simulink model "v13_aa_rii_16r128dr_ver104"  (Simulink 7.9)
settings: Solver=FixedStepDiscrete  SolverMode=SingleTasking  StartTime=0.0  StopTime=32768  RelTol=1e-3
undefined symbols (not defined in the file or in its folder): sfp
libraries: xbsIndex_r4 x19795, xps_library x54, casper_library_misc x36, dspsigops x32
(part 6 of 212)

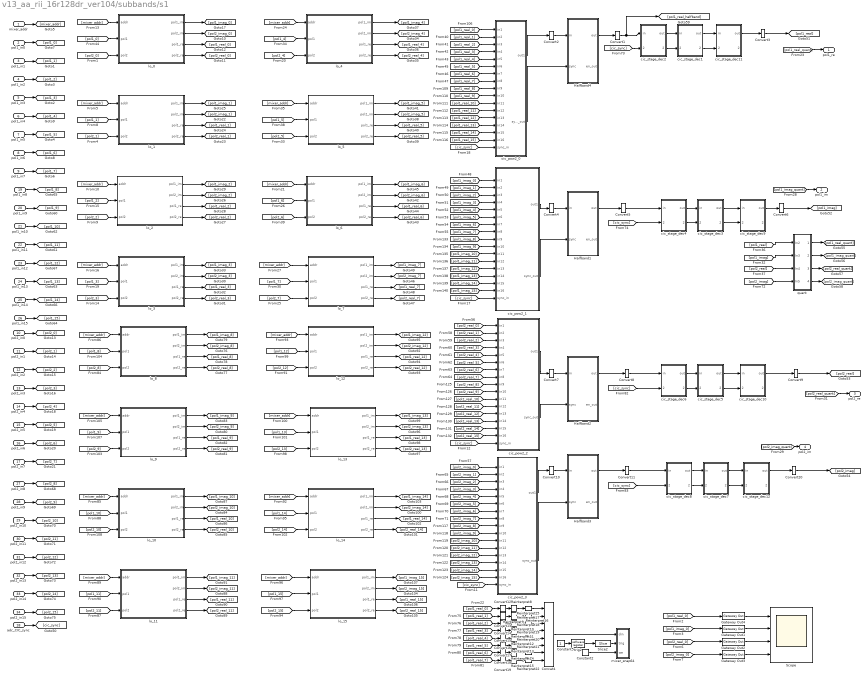
== system v13_aa_rii_16r128dr_ver104/subbands/s1 ==
  mixer_addr : Inport
  pol1_in0 : Inport  Port=2
  pol1_in1 : Inport  Port=3
  pol1_in2 : Inport  Port=4
  pol1_in3 : Inport  Port=5
  pol1_in4 : Inport  Port=6
  pol1_in5 : Inport  Port=7
  pol1_in6 : Inport  Port=8
  pol1_in7 : Inport  Port=9
  pol2_in0 : Inport  Port=10
  pol2_in1 : Inport  Port=11
  pol2_in2 : Inport  Port=12
  pol2_in3 : Inport  Port=13
  pol2_in4 : Inport  Port=14
  pol2_in5 : Inport  Port=15
  pol2_in6 : Inport  Port=16
  pol2_in7 : Inport  Port=17
  adc_CIC_sync : Inport  Port=18
  pol1_in8 : Inport  Port=19
  pol1_in9 : Inport  Port=20
  pol1_in10 : Inport  Port=21
  pol1_in11 : Inport  Port=22
  pol1_in12 : Inport  Port=23
  pol1_in13 : Inport  Port=24
  pol1_in14 : Inport  Port=25
  pol1_in15 : Inport  Port=26
  pol2_in8 : Inport  Port=27
  pol2_in9 : Inport  Port=28
  pol2_in10 : Inport  Port=29
  pol2_in11 : Inport  Port=30
  pol2_in12 : Inport  Port=31
  pol2_in13 : Inport  Port=32
  pol2_in14 : Inport  Port=33
  pol2_in15 : Inport  Port=34
  Concat4 : xbsIndex_r4/Concat  num_inputs=8  dbl_ovrd=off  block_type=concat
  Constant2 : xbsIndex_r4/Constant  const=1  gui_display_data_type=Boolean  arith_type=Boolean  n_bits=16  bin_pt=14  preci_type=Single  exp_width=8  frac_width=24  explicit_period=off  period=1  equ=P=C  opselect=C  inp2=PCIN>>17  opr=+  inp1=P  carry=CIN  dbl_ovrd=off  block_type=constant
  Constant5 : Constant
  Convert{1,5,8,11} (4 alike) : xbsIndex_r4/Convert  gui_display_data_type=Fixed-point  arith_type="Signed  (2's comp)"  n_bits=32  bin_pt=12  float_type=Single  exp_bits=8  fraction_bits=24  quantization=Truncate  overflow=Wrap  en=off  latency=1  dbl_ovrd=off  pipeline=off  block_type=convert
  Convert{2,4,7,10} (4 alike) : xbsIndex_r4/Convert  gui_display_data_type=Fixed-point  arith_type="Signed  (2's comp)"  n_bits=32  bin_pt=18  float_type=Single  exp_bits=8  fraction_bits=24  quantization=Truncate  overflow=Wrap  en=off  latency=1  dbl_ovrd=off  pipeline=off  block_type=convert
  Convert{12..19} (8 alike) : xbsIndex_r4/Convert  gui_display_data_type=Fixed-point  arith_type=Unsigned  n_bits=8  bin_pt=6  float_type=Single  exp_bits=8  fraction_bits=24  quantization=Truncate  overflow=Wrap  en=off  latency=2  dbl_ovrd=off  pipeline=off  block_type=convert
      settings: block_version=11.4
  Convert{3,6,9,20} (4 alike) : xbsIndex_r4/Convert  gui_display_data_type=Fixed-point  arith_type="Signed  (2's comp)"  n_bits=32  bin_pt=6  float_type=Single  exp_bits=8  fraction_bits=24  quantization=Truncate  overflow=Wrap  en=off  latency=1  dbl_ovrd=off  pipeline=off  block_type=convert
  Gateway Out{1..4} (4 alike) : xbsIndex_r4/Gateway Out  inherit_from_input=off  hdl_port=on  timing_constraint=None  locs_specified=off  LOCs={}  UseAsDAC=off  DACChannel='1'  block_type=gatewayout
      settings: infoedit="Gateway out block.  Converts Xilinx fixed point or floating-point type inputs into ouputs of type Simulink integer, double, or fixed point.<br><br>Hardware notes:  In hardware these blocks become top level output ports or are discarded, depending on how they are configured."
  Halfband1 : Subsystem  AncestorBlock=gbt_library/Halfband  mask(Halfband FIR){mult_latency=3, add_latency=2, hold_period=1, passband=0.4, fir_len=7}
      mask prompts: mult_latency "Multiplication latency"; add_latency "Add latency"; hold_period "Hold Period"; passband "Width of Halfband passband (<0.5)"; fir_len "FIR Length (odd) [Number of Taps will be: 2*fir_len+1]"
      mask help: A halfband FIR for low rate computation.
      mask init:
      | coeffs = halfband_coeff_gen(fir_len, passband);
      | config.source=str2func('hold_fir_init_xblock');
      | config.depend=[get_dependlist('hold_fir'),{'halfband_coeff_gen'}];
      | config.toplevel=gcb;
      | set_param(gcb,'LinkStatus','inactive');
      | xBlock(config, {config.toplevel,...
      |     'mult_latency', mult_latency, ...
      |     'add_latency', add_latency, ...
      |     'hold_period', hold_period, ...
      |     'coeffs', coeffs});
      settings: AttributesFormatString=|
      | 15-tap FIR filter (9 nonzero tap)
      |  hold period: 1
      |  mult latency: 3
      |  add latency: 2
  Halfband2 : Subsystem  AncestorBlock=gbt_library/Halfband  mask(Halfband FIR){mult_latency=3, add_latency=2, hold_period=1, passband=0.4, fir_len=7}
      mask prompts: mult_latency "Multiplication latency"; add_latency "Add latency"; hold_period "Hold Period"; passband "Width of Halfband passband (<0.5)"; fir_len "FIR Length (odd) [Number of Taps will be: 2*fir_len+1]"
      mask help: A halfband FIR for low rate computation.
      mask init: same as v13_aa_rii_16r128dr_ver104/subbands/s1/Halfband1
      settings: AttributesFormatString=|
      | 15-tap FIR filter (9 nonzero tap)
      |  hold period: 1
      |  mult latency: 3
      |  add latency: 2
  Halfband3 : Subsystem  AncestorBlock=gbt_library/Halfband  mask(Halfband FIR){mult_latency=3, add_latency=2, hold_period=1, passband=0.4, fir_len=7}
      mask prompts: mult_latency "Multiplication latency"; add_latency "Add latency"; hold_period "Hold Period"; passband "Width of Halfband passband (<0.5)"; fir_len "FIR Length (odd) [Number of Taps will be: 2*fir_len+1]"
      mask help: A halfband FIR for low rate computation.
      mask init: same as v13_aa_rii_16r128dr_ver104/subbands/s1/Halfband1
      settings: AttributesFormatString=|
      | 15-tap FIR filter (9 nonzero tap)
      |  hold period: 1
      |  mult latency: 3
      |  add latency: 2
  Halfband4 : Subsystem  AncestorBlock=gbt_library/Halfband  mask(Halfband FIR){mult_latency=3, add_latency=2, hold_period=1, passband=0.4, fir_len=7}
      mask prompts: mult_latency "Multiplication latency"; add_latency "Add latency"; hold_period "Hold Period"; passband "Width of Halfband passband (<0.5)"; fir_len "FIR Length (odd) [Number of Taps will be: 2*fir_len+1]"
      mask help: A halfband FIR for low rate computation.
      mask init: same as v13_aa_rii_16r128dr_ver104/subbands/s1/Halfband1
      settings: AttributesFormatString=|
      | 15-tap FIR filter (9 nonzero tap)
      |  hold period: 1
      |  mult latency: 3
      |  add latency: 2
  Reinterpret{8..15} (8 alike) : xbsIndex_r4/Reinterpret  force_arith_type=on  arith_type="Signed  (2's comp)"  force_bin_pt=on  bin_pt=7  block_type=reinterpret
  Reinterpret{16..22,25} (8 alike) : xbsIndex_r4/Reinterpret  force_arith_type=on  arith_type=Unsigned  force_bin_pt=on  bin_pt=0  block_type=reinterpret
  Scope : Scope  NumInputPorts=4
      settings: YMin=-0.0075~-1~-0.0075~-1  YMax=0.0075~1~0.0075~1
  Slice2 : xbsIndex_r4/Slice  nbits=1  boolean_output=on  mode=Lower Bit Location + Width  bit1=0  base1=MSB of Input  bit0=0  base0=LSB of Input  dbl_ovrd=off  block_type=slice
  cic_pow2_0 : Subsystem  AncestorBlock=gbt_library/cic_pow2  mask(CIC (pow2)){n_stages=4, dec_rate=16, input_clk_period=1, n_inputs=16, add_latency=1, n_bits=8, bin_pt=7}
      mask prompts: n_stages "CIC filter Order"; dec_rate "Decimation rate"; input_clk_period "Input Clock Period"; n_inputs "Number of inputs"; add_latency "Add latency"; n_bits "Input bit width"; bin_pt "Binary point"
      mask help:
      | A non-recursive CIC filter with parallel inputs.
      | Supports decimation rate of power of 2.
      | A multi-stage implementation with each stage of decimation rate 2.
      mask init:
      | config.source = str2func('cic_pow2_init_xblock');
      | config.toplevel = gcb;
      | config.depend = get_dependlist('cic_pow2'); 
      | set_param(gcb,'LinkStatus','inactive');
      | xBlock(config, {config.toplevel,...
      |                 'n_stages',n_stages, ...
      |                 'dec_rate', dec_rate, ...
      |                 'input_clk_rate', input_clk_period, ...
      |                 'n_inputs', n_inputs,...
      |                 'add_latency',add_latency,...
      |                 'n_bits', n_bits, ...
      |                 'bin_pt', bin_pt} ...
      |                 );
      | disp('new block added');
      settings: AttributesFormatString=|
      | dec_rate = 16
      | Order = 4
  cic_pow2_1 : Subsystem  AncestorBlock=gbt_library/cic_pow2  mask(CIC (pow2)){n_stages=4, dec_rate=16, input_clk_period=1, n_inputs=16, add_latency=1, n_bits=8, bin_pt=7}
      mask prompts: n_stages "CIC filter Order"; dec_rate "Decimation rate"; input_clk_period "Input Clock Period"; n_inputs "Number of inputs"; add_latency "Add latency"; n_bits "Input bit width"; bin_pt "Binary point"
      mask help:
      | A non-recursive CIC filter with parallel inputs.
      | Supports decimation rate of power of 2.
      | A multi-stage implementation with each stage of decimation rate 2.
      mask init: same as v13_aa_rii_16r128dr_ver104/subbands/s1/cic_pow2_0
      settings: AttributesFormatString=|
      | dec_rate = 16
      | Order = 4
  cic_pow2_2 : Subsystem  AncestorBlock=gbt_library/cic_pow2  mask(CIC (pow2)){n_stages=4, dec_rate=16, input_clk_period=1, n_inputs=16, add_latency=1, n_bits=8, bin_pt=7}
      mask prompts: n_stages "CIC filter Order"; dec_rate "Decimation rate"; input_clk_period "Input Clock Period"; n_inputs "Number of inputs"; add_latency "Add latency"; n_bits "Input bit width"; bin_pt "Binary point"
      mask help:
      | A non-recursive CIC filter with parallel inputs.
      | Supports decimation rate of power of 2.
      | A multi-stage implementation with each stage of decimation rate 2.
      mask init: same as v13_aa_rii_16r128dr_ver104/subbands/s1/cic_pow2_0
      settings: AttributesFormatString=|
      | dec_rate = 16
      | Order = 4
  cic_pow2_3 : Subsystem  AncestorBlock=gbt_library/cic_pow2  mask(CIC (pow2)){n_stages=4, dec_rate=16, input_clk_period=1, n_inputs=16, add_latency=1, n_bits=8, bin_pt=7}
      mask prompts: n_stages "CIC filter Order"; dec_rate "Decimation rate"; input_clk_period "Input Clock Period"; n_inputs "Number of inputs"; add_latency "Add latency"; n_bits "Input bit width"; bin_pt "Binary point"
      mask help:
      | A non-recursive CIC filter with parallel inputs.
      | Supports decimation rate of power of 2.
      | A multi-stage implementation with each stage of decimation rate 2.
      mask init: same as v13_aa_rii_16r128dr_ver104/subbands/s1/cic_pow2_0
      settings: AttributesFormatString=|
      | dec_rate = 16
      | Order = 4
  cic_stage_dec1 : Subsystem  AncestorBlock=gbt_library/cic_stage_dec2  mask(CIC stage (dec2)){n_stages=3, recursive=off, dec_rate=2, n_inputs=1, input_clk_period=1, input_period=2, add_latency=2, n_bits=8, bin_pt=7}
      mask prompts: n_stages "CIC filter Order"; recursive "Recursive?"; dec_rate "Decimation rate"; n_inputs "Number of Inputs"; input_clk_period "Input Clock period (Actual)"; input_period "Effective Input Sampling Period"; add_latency "Add latency"; n_bits "Input bit width"; bin_pt "Binary point"
      mask help:
      | A single stage of CIC filter with even number of parallel inputs.
      | Only supports decimation rate = 2.
      | If n_inputs > 1, number of outputs = n_inputs/2.
      | Non-recursive.
      |
      | Clock period:
      | input_clk_period is the actual sampling period of incoming data stream.
      | input_period is the effective (or expected) sampling period of incoming data stream.
      | E.g. For incoming data stream X[n] = [1& 1 2 2 3 3 4 4 5 5 ...], the input_clk_period is 1 and the input_period is 2.
      mask init:
      | if recursive
      |     % recursive case
      |     config.source=str2func('cic_stage_dec2_recursive_init_xblock');
      |     config.toplevel=gcb;
      |     config.depend = get_dependlist('cic_stage_dec2_recursive');
      |     set_param(gcb,'LinkStatus','inactive');
      |     xBlock(config, {config.toplevel, ...
      |                 'n_stages', n_stages, ...
      |                 'dec_rate', dec_rate, ...
      |                 'n_inputs', n_inputs, ...
      |                 'input_clk_period', input_clk_period, ...
      |                 'input_period', input_period, ...
      |                 'add_latency', add_latency, ...
      |                 'input_bits', n_bits, ...
      |                 'bin_pt', bin_pt, ...
      |                 'delay_len', 1});
      | else
      |     % non-recursive case
      |     config.source=str2func('cic_stage_dec2_init_xblock');
      |     config.toplevel=gcb;
      |     config.depend = get_dependlist('cic_stage_dec2');
      |     set_param(gcb,'LinkStatus','inactive');
      |     xBlock(config, {config.toplevel, ...
      |                 'n_stages', n_stages, ...
      |                 'dec_rate', dec_rate, ...
      |                 'n_inputs', n_inputs, ...
      |                 'input_clk_period', input_clk_period, ...
      |                 'input_period', input_period, ...
      |                 'add_latency', add_latency, ...
      |                 'n_bits', n_bits, ...
      |                 'bin_pt', bin_pt});
      | end
      settings: AttributesFormatString=|
      | nonrecursive
      | 8_7_in
      | clock(actualy/effective): 1/2
      mask CallbackString:
      | ||n=get_param(gcb,'dec_rate');
      | if ~isempty(n)
      |     n = eval(n);
      |     if ~isprime(n) && n~=1
      |         errordlg('Only supports decimation rate that is a prime number or 1');
      |         set_param(gcb,'dec_rate','');
      |     end
      | end||||||
  cic_stage_dec10 : Subsystem  AncestorBlock=gbt_library/cic_stage_dec2  mask(CIC stage (dec2)){n_stages=3, recursive=off, dec_rate=2, n_inputs=1, input_clk_period=1, input_period=4, add_latency=2, n_bits=8, bin_pt=7}
      mask prompts: n_stages "CIC filter Order"; recursive "Recursive?"; dec_rate "Decimation rate"; n_inputs "Number of Inputs"; input_clk_period "Input Clock period (Actual)"; input_period "Effective Input Sampling Period"; add_latency "Add latency"; n_bits "Input bit width"; bin_pt "Binary point"
      mask help:
      | A single stage of CIC filter with even number of parallel inputs.
      | Only supports decimation rate = 2.
      | If n_inputs > 1, number of outputs = n_inputs/2.
      | Non-recursive.
      |
      | Clock period:
      | input_clk_period is the actual sampling period of incoming data stream.
      | input_period is the effective (or expected) sampling period of incoming data stream.
      | E.g. For incoming data stream X[n] = [1& 1 2 2 3 3 4 4 5 5 ...], the input_clk_period is 1 and the input_period is 2.
      mask init: same as v13_aa_rii_16r128dr_ver104/subbands/s1/cic_stage_dec1
      settings: AttributesFormatString=|
      | nonrecursive
      | 8_7_in
      | clock(actualy/effective): 1/4
      mask CallbackString:
      | ||n=get_param(gcb,'dec_rate');
      | if ~isempty(n)
      |     n = eval(n);
      |     if ~isprime(n) && n~=1
      |         errordlg('Only supports decimation rate that is a prime number or 1');
      |         set_param(gcb,'dec_rate','');
      |     end
      | end||||||
  cic_stage_dec11 : Subsystem  AncestorBlock=gbt_library/cic_stage_dec2  mask(CIC stage (dec2)){n_stages=3, recursive=off, dec_rate=2, n_inputs=1, input_clk_period=1, input_period=4, add_latency=2, n_bits=8, bin_pt=7}
      mask prompts: n_stages "CIC filter Order"; recursive "Recursive?"; dec_rate "Decimation rate"; n_inputs "Number of Inputs"; input_clk_period "Input Clock period (Actual)"; input_period "Effective Input Sampling Period"; add_latency "Add latency"; n_bits "Input bit width"; bin_pt "Binary point"
      mask help:
      | A single stage of CIC filter with even number of parallel inputs.
      | Only supports decimation rate = 2.
      | If n_inputs > 1, number of outputs = n_inputs/2.
      | Non-recursive.
      |
      | Clock period:
      | input_clk_period is the actual sampling period of incoming data stream.
      | input_period is the effective (or expected) sampling period of incoming data stream.
      | E.g. For incoming data stream X[n] = [1& 1 2 2 3 3 4 4 5 5 ...], the input_clk_period is 1 and the input_period is 2.
      mask init: same as v13_aa_rii_16r128dr_ver104/subbands/s1/cic_stage_dec1
      settings: AttributesFormatString=|
      | nonrecursive
      | 8_7_in
      | clock(actualy/effective): 1/4
      mask CallbackString:
      | ||n=get_param(gcb,'dec_rate');
      | if ~isempty(n)
      |     n = eval(n);
      |     if ~isprime(n) && n~=1
      |         errordlg('Only supports decimation rate that is a prime number or 1');
      |         set_param(gcb,'dec_rate','');
      |     end
      | end||||||
  cic_stage_dec12 : Subsystem  AncestorBlock=gbt_library/cic_stage_dec2  mask(CIC stage (dec2)){n_stages=3, recursive=off, dec_rate=2, n_inputs=1, input_clk_period=1, input_period=4, add_latency=2, n_bits=8, bin_pt=7}
      mask prompts: n_stages "CIC filter Order"; recursive "Recursive?"; dec_rate "Decimation rate"; n_inputs "Number of Inputs"; input_clk_period "Input Clock period (Actual)"; input_period "Effective Input Sampling Period"; add_latency "Add latency"; n_bits "Input bit width"; bin_pt "Binary point"
      mask help:
      | A single stage of CIC filter with even number of parallel inputs.
      | Only supports decimation rate = 2.
      | If n_inputs > 1, number of outputs = n_inputs/2.
      | Non-recursive.
      |
      | Clock period:
      | input_clk_period is the actual sampling period of incoming data stream.
      | input_period is the effective (or expected) sampling period of incoming data stream.
      | E.g. For incoming data stream X[n] = [1& 1 2 2 3 3 4 4 5 5 ...], the input_clk_period is 1 and the input_period is 2.
      mask init: same as v13_aa_rii_16r128dr_ver104/subbands/s1/cic_stage_dec1
      settings: AttributesFormatString=|
      | nonrecursive
      | 8_7_in
      | clock(actualy/effective): 1/4
      mask CallbackString:
      | ||n=get_param(gcb,'dec_rate');
      | if ~isempty(n)
      |     n = eval(n);
      |     if ~isprime(n) && n~=1
      |         errordlg('Only supports decimation rate that is a prime number or 1');
      |         set_param(gcb,'dec_rate','');
      |     end
      | end||||||
  cic_stage_dec2 : Subsystem  AncestorBlock=gbt_library/cic_stage_dec2  mask(CIC stage (dec2)){n_stages=3, recursive=off, dec_rate=2, n_inputs=1, input_clk_period=1, input_period=1, add_latency=2, n_bits=8, bin_pt=7}
      mask prompts: n_stages "CIC filter Order"; recursive "Recursive?"; dec_rate "Decimation rate"; n_inputs "Number of Inputs"; input_clk_period "Input Clock period (Actual)"; input_period "Effective Input Sampling Period"; add_latency "Add latency"; n_bits "Input bit width"; bin_pt "Binary point"
      mask help:
      | A single stage of CIC filter with even number of parallel inputs.
      | Only supports decimation rate = 2.
      | If n_inputs > 1, number of outputs = n_inputs/2.
      | Non-recursive.
      |
      | Clock period:
      | input_clk_period is the actual sampling period of incoming data stream.
      | input_period is the effective (or expected) sampling period of incoming data stream.
      | E.g. For incoming data stream X[n] = [1& 1 2 2 3 3 4 4 5 5 ...], the input_clk_period is 1 and the input_period is 2.
      mask init: same as v13_aa_rii_16r128dr_ver104/subbands/s1/cic_stage_dec1
      settings: AttributesFormatString=|
      | nonrecursive
      | 8_7_in
      | clock(actualy/effective): 1/1
      mask CallbackString:
      | ||n=get_param(gcb,'dec_rate');
      | if ~isempty(n)
      |     n = eval(n);
      |     if ~isprime(n) && n~=1
      |         errordlg('Only supports decimation rate that is a prime number or 1');
      |         set_param(gcb,'dec_rate','');
      |     end
      | end||||||
  cic_stage_dec3 : Subsystem  AncestorBlock=gbt_library/cic_stage_dec2  mask(CIC stage (dec2)){n_stages=3, recursive=off, dec_rate=2, n_inputs=1, input_clk_period=1, input_period=2, add_latency=2, n_bits=8, bin_pt=7}
      mask prompts: n_stages "CIC filter Order"; recursive "Recursive?"; dec_rate "Decimation rate"; n_inputs "Number of Inputs"; input_clk_period "Input Clock period (Actual)"; input_period "Effective Input Sampling Period"; add_latency "Add latency"; n_bits "Input bit width"; bin_pt "Binary point"
      mask help:
      | A single stage of CIC filter with even number of parallel inputs.
      | Only supports decimation rate = 2.
      | If n_inputs > 1, number of outputs = n_inputs/2.
      | Non-recursive.
      |
      | Clock period:
      | input_clk_period is the actual sampling period of incoming data stream.
      | input_period is the effective (or expected) sampling period of incoming data stream.
      | E.g. For incoming data stream X[n] = [1& 1 2 2 3 3 4 4 5 5 ...], the input_clk_period is 1 and the input_period is 2.
      mask init: same as v13_aa_rii_16r128dr_ver104/subbands/s1/cic_stage_dec1
      settings: AttributesFormatString=|
      | nonrecursive
      | 8_7_in
      | clock(actualy/effective): 1/2
      mask CallbackString:
      | ||n=get_param(gcb,'dec_rate');
      | if ~isempty(n)
      |     n = eval(n);
      |     if ~isprime(n) && n~=1
      |         errordlg('Only supports decimation rate that is a prime number or 1');
      |         set_param(gcb,'dec_rate','');
      |     end
      | end||||||
  cic_stage_dec4 : Subsystem  AncestorBlock=gbt_library/cic_stage_dec2  mask(CIC stage (dec2)){n_stages=3, recursive=off, dec_rate=2, n_inputs=1, input_clk_period=1, input_period=1, add_latency=2, n_bits=8, bin_pt=7}
      mask prompts: n_stages "CIC filter Order"; recursive "Recursive?"; dec_rate "Decimation rate"; n_inputs "Number of Inputs"; input_clk_period "Input Clock period (Actual)"; input_period "Effective Input Sampling Period"; add_latency "Add latency"; n_bits "Input bit width"; bin_pt "Binary point"
      mask help:
      | A single stage of CIC filter with even number of parallel inputs.
      | Only supports decimation rate = 2.
      | If n_inputs > 1, number of outputs = n_inputs/2.
      | Non-recursive.
      |
      | Clock period:
      | input_clk_period is the actual sampling period of incoming data stream.
      | input_period is the effective (or expected) sampling period of incoming data stream.
      | E.g. For incoming data stream X[n] = [1& 1 2 2 3 3 4 4 5 5 ...], the input_clk_period is 1 and the input_period is 2.
      mask init: same as v13_aa_rii_16r128dr_ver104/subbands/s1/cic_stage_dec1
      settings: AttributesFormatString=|
      | nonrecursive
      | 8_7_in
      | clock(actualy/effective): 1/1
      mask CallbackString:
      | ||n=get_param(gcb,'dec_rate');
      | if ~isempty(n)
      |     n = eval(n);
      |     if ~isprime(n) && n~=1
      |         errordlg('Only supports decimation rate that is a prime number or 1');
      |         set_param(gcb,'dec_rate','');
      |     end
      | end||||||
  cic_stage_dec5 : Subsystem  AncestorBlock=gbt_library/cic_stage_dec2  mask(CIC stage (dec2)){n_stages=3, recursive=off, dec_rate=2, n_inputs=1, input_clk_period=1, input_period=2, add_latency=2, n_bits=8, bin_pt=7}
      mask prompts: n_stages "CIC filter Order"; recursive "Recursive?"; dec_rate "Decimation rate"; n_inputs "Number of Inputs"; input_clk_period "Input Clock period (Actual)"; input_period "Effective Input Sampling Period"; add_latency "Add latency"; n_bits "Input bit width"; bin_pt "Binary point"
      mask help:
      | A single stage of CIC filter with even number of parallel inputs.
      | Only supports decimation rate = 2.
      | If n_inputs > 1, number of outputs = n_inputs/2.
      | Non-recursive.
      |
      | Clock period:
      | input_clk_period is the actual sampling period of incoming data stream.
      | input_period is the effective (or expected) sampling period of incoming data stream.
      | E.g. For incoming data stream X[n] = [1& 1 2 2 3 3 4 4 5 5 ...], the input_clk_period is 1 and the input_period is 2.
      mask init: same as v13_aa_rii_16r128dr_ver104/subbands/s1/cic_stage_dec1
      settings: AttributesFormatString=|
      | nonrecursive
      | 8_7_in
      | clock(actualy/effective): 1/2
      mask CallbackString:
      | ||n=get_param(gcb,'dec_rate');
      | if ~isempty(n)
      |     n = eval(n);
      |     if ~isprime(n) && n~=1
      |         errordlg('Only supports decimation rate that is a prime number or 1');
      |         set_param(gcb,'dec_rate','');
      |     end
      | end||||||
  cic_stage_dec6 : Subsystem  AncestorBlock=gbt_library/cic_stage_dec2  mask(CIC stage (dec2)){n_stages=3, recursive=off, dec_rate=2, n_inputs=1, input_clk_period=1, input_period=1, add_latency=2, n_bits=8, bin_pt=7}
      mask prompts: n_stages "CIC filter Order"; recursive "Recursive?"; dec_rate "Decimation rate"; n_inputs "Number of Inputs"; input_clk_period "Input Clock period (Actual)"; input_period "Effective Input Sampling Period"; add_latency "Add latency"; n_bits "Input bit width"; bin_pt "Binary point"
      mask help:
      | A single stage of CIC filter with even number of parallel inputs.
      | Only supports decimation rate = 2.
      | If n_inputs > 1, number of outputs = n_inputs/2.
      | Non-recursive.
      |
      | Clock period:
      | input_clk_period is the actual sampling period of incoming data stream.
      | input_period is the effective (or expected) sampling period of incoming data stream.
      | E.g. For incoming data stream X[n] = [1& 1 2 2 3 3 4 4 5 5 ...], the input_clk_period is 1 and the input_period is 2.
      mask init: same as v13_aa_rii_16r128dr_ver104/subbands/s1/cic_stage_dec1
      settings: AttributesFormatString=|
      | nonrecursive
      | 8_7_in
      | clock(actualy/effective): 1/1
      mask CallbackString:
      | ||n=get_param(gcb,'dec_rate');
      | if ~isempty(n)
      |     n = eval(n);
      |     if ~isprime(n) && n~=1
      |         errordlg('Only supports decimation rate that is a prime number or 1');
      |         set_param(gcb,'dec_rate','');
      |     end
      | end||||||
  cic_stage_dec7 : Subsystem  AncestorBlock=gbt_library/cic_stage_dec2  mask(CIC stage (dec2)){n_stages=3, recursive=off, dec_rate=2, n_inputs=1, input_clk_period=1, input_period=2, add_latency=2, n_bits=8, bin_pt=7}
      mask prompts: n_stages "CIC filter Order"; recursive "Recursive?"; dec_rate "Decimation rate"; n_inputs "Number of Inputs"; input_clk_period "Input Clock period (Actual)"; input_period "Effective Input Sampling Period"; add_latency "Add latency"; n_bits "Input bit width"; bin_pt "Binary point"
      mask help:
      | A single stage of CIC filter with even number of parallel inputs.
      | Only supports decimation rate = 2.
      | If n_inputs > 1, number of outputs = n_inputs/2.
      | Non-recursive.
      |
      | Clock period:
      | input_clk_period is the actual sampling period of incoming data stream.
      | input_period is the effective (or expected) sampling period of incoming data stream.
      | E.g. For incoming data stream X[n] = [1& 1 2 2 3 3 4 4 5 5 ...], the input_clk_period is 1 and the input_period is 2.
      mask init: same as v13_aa_rii_16r128dr_ver104/subbands/s1/cic_stage_dec1
      settings: AttributesFormatString=|
      | nonrecursive
      | 8_7_in
      | clock(actualy/effective): 1/2
      mask CallbackString:
      | ||n=get_param(gcb,'dec_rate');
      | if ~isempty(n)
      |     n = eval(n);
      |     if ~isprime(n) && n~=1
      |         errordlg('Only supports decimation rate that is a prime number or 1');
      |         set_param(gcb,'dec_rate','');
      |     end
      | end||||||
  cic_stage_dec8 : Subsystem  AncestorBlock=gbt_library/cic_stage_dec2  mask(CIC stage (dec2)){n_stages=3, recursive=off, dec_rate=2, n_inputs=1, input_clk_period=1, input_period=1, add_latency=2, n_bits=8, bin_pt=7}
      mask prompts: n_stages "CIC filter Order"; recursive "Recursive?"; dec_rate "Decimation rate"; n_inputs "Number of Inputs"; input_clk_period "Input Clock period (Actual)"; input_period "Effective Input Sampling Period"; add_latency "Add latency"; n_bits "Input bit width"; bin_pt "Binary point"
      mask help:
      | A single stage of CIC filter with even number of parallel inputs.
      | Only supports decimation rate = 2.
      | If n_inputs > 1, number of outputs = n_inputs/2.
      | Non-recursive.
      |
      | Clock period:
      | input_clk_period is the actual sampling period of incoming data stream.
      | input_period is the effective (or expected) sampling period of incoming data stream.
      | E.g. For incoming data stream X[n] = [1& 1 2 2 3 3 4 4 5 5 ...], the input_clk_period is 1 and the input_period is 2.
      mask init: same as v13_aa_rii_16r128dr_ver104/subbands/s1/cic_stage_dec1
      settings: AttributesFormatString=|
      | nonrecursive
      | 8_7_in
      | clock(actualy/effective): 1/1
      mask CallbackString:
      | ||n=get_param(gcb,'dec_rate');
      | if ~isempty(n)
      |     n = eval(n);
      |     if ~isprime(n) && n~=1
      |         errordlg('Only supports decimation rate that is a prime number or 1');
      |         set_param(gcb,'dec_rate','');
      |     end
      | end||||||
  cic_stage_dec9 : Subsystem  AncestorBlock=gbt_library/cic_stage_dec2  mask(CIC stage (dec2)){n_stages=3, recursive=off, dec_rate=2, n_inputs=1, input_clk_period=1, input_period=4, add_latency=2, n_bits=8, bin_pt=7}
      mask prompts: n_stages "CIC filter Order"; recursive "Recursive?"; dec_rate "Decimation rate"; n_inputs "Number of Inputs"; input_clk_period "Input Clock period (Actual)"; input_period "Effective Input Sampling Period"; add_latency "Add latency"; n_bits "Input bit width"; bin_pt "Binary point"
      mask help:
      | A single stage of CIC filter with even number of parallel inputs.
      | Only supports decimation rate = 2.
      | If n_inputs > 1, number of outputs = n_inputs/2.
      | Non-recursive.
      |
      | Clock period:
      | input_clk_period is the actual sampling period of incoming data stream.
      | input_period is the effective (or expected) sampling period of incoming data stream.
      | E.g. For incoming data stream X[n] = [1& 1 2 2 3 3 4 4 5 5 ...], the input_clk_period is 1 and the input_period is 2.
      mask init: same as v13_aa_rii_16r128dr_ver104/subbands/s1/cic_stage_dec1
      settings: AttributesFormatString=|
      | nonrecursive
      | 8_7_in
      | clock(actualy/effective): 1/4
      mask CallbackString:
      | ||n=get_param(gcb,'dec_rate');
      | if ~isempty(n)
      |     n = eval(n);
      |     if ~isprime(n) && n~=1
      |         errordlg('Only supports decimation rate that is a prime number or 1');
      |         set_param(gcb,'dec_rate','');
      |     end
      | end||||||
  lo_0 : Subsystem  AncestorBlock=gbt_library/complex_mix_lo
  lo_1 : Subsystem  AncestorBlock=gbt_library/complex_mix_lo
  lo_10 : Subsystem  AncestorBlock=gbt_library/complex_mix_lo
  lo_11 : Subsystem  AncestorBlock=gbt_library/complex_mix_lo
  lo_12 : Subsystem  AncestorBlock=gbt_library/complex_mix_lo
  lo_13 : Subsystem  AncestorBlock=gbt_library/complex_mix_lo
  lo_14 : Subsystem  AncestorBlock=gbt_library/complex_mix_lo
  lo_15 : Subsystem  AncestorBlock=gbt_library/complex_mix_lo
  lo_2 : Subsystem  AncestorBlock=gbt_library/complex_mix_lo
  lo_3 : Subsystem  AncestorBlock=gbt_library/complex_mix_lo
  lo_4 : Subsystem  AncestorBlock=gbt_library/complex_mix_lo
  lo_5 : Subsystem  AncestorBlock=gbt_library/complex_mix_lo
  lo_6 : Subsystem  AncestorBlock=gbt_library/complex_mix_lo
  lo_7 : Subsystem  AncestorBlock=gbt_library/complex_mix_lo
  lo_8 : Subsystem  AncestorBlock=gbt_library/complex_mix_lo
  lo_9 : Subsystem  AncestorBlock=gbt_library/complex_mix_lo
  mixer_snap64 : Subsystem  AncestorBlock=casper_library_scopes/snap64  mask(snap64){nsamples=11}
      mask prompts: nsamples "No. of samples(2^?)"
      mask help:
      | The <i>snap</i> block provides a packaged solution to capturing data from
      | the FPGA fabric and making it accessible from the CPU.  The block comes in
      | 2 flavors: <i>snap</i> (which captures to a 32 bit wide Shared BRAM), and
      | <i>snap64</i> (which captures to 2x32 bit wide Shared BRAMs to effect a
      | 64 bit capture).
      mask Help: eval('xlWeb(''http://casper.berkeley.edu/wiki/Snap'')')
  quant : Subsystem
  trig2 : xps_library/software register  Tag=xps:sw_reg  io_dir=From Processor  arith_type=Unsigned  bitwidth=32  bin_pt=0  sample_period=1  gw_name=v13_aa_rii_16r128dr_ver104_subbands_s1_trig2_user_data_out
      link override cic_filter_testing_to_compile_trig_user_data_out: arith_type=Unsigned  n_bits=32  bin_pt=0  period=1
      link override newmode13_subband1_trig2_user_data_out: arith_type=Unsigned  n_bits=32  bin_pt=0  period=1
  pol1_re : Outport
  pol1_im : Outport  Port=2
  pol2_re : Outport  Port=3
  pol2_im : Outport  Port=4
  wires:
  mixer_addr/1 -> [mixer_addr]
  pol1_in0/1 -> [pol1_0]
  pol1_in1/1 -> [pol1_1]
  pol1_in2/1 -> [pol1_2]
  pol1_in3/1 -> [pol1_3]
  pol1_in4/1 -> [pol1_4]
  pol1_in5/1 -> [pol1_5]
  pol1_in6/1 -> [pol1_6]
  pol1_in7/1 -> [pol1_7]
  pol2_in0/1 -> [pol2_0]
  pol2_in1/1 -> [pol2_1]
  pol2_in2/1 -> [pol2_2]
  pol2_in3/1 -> [pol2_3]
  pol2_in4/1 -> [pol2_4]
  pol2_in5/1 -> [pol2_5]
  pol2_in6/1 -> [pol2_6]
  pol2_in7/1 -> [pol2_7]
  [mixer_addr] -> lo_1/1(addr)
  [mixer_addr] -> lo_2/1(addr)
  [mixer_addr] -> lo_3/1(addr)
  lo_4/1(pol1_im) -> [pol1_imag_4]
  lo_4/2(pol2_im) -> [pol2_imag_4]
  lo_4/3(pol1_re) -> [pol1_real_4]
  lo_4/4(pol2_re) -> [pol2_real_4]
  [mixer_addr] -> lo_4/1(addr)
  [pol2_4] -> lo_4/3(pol2)
  [pol1_4] -> lo_4/2(pol1)
  [mixer_addr] -> lo_0/1(addr)
  [pol1_0] -> lo_0/2(pol1)
  [pol2_0] -> lo_0/3(pol2)
  lo_0/1(pol1_im) -> [pol1_imag_0]
  lo_0/2(pol2_im) -> [pol2_imag_0]
  lo_0/3(pol1_re) -> [pol1_real_0]
  lo_0/4(pol2_re) -> [pol2_real_0]
  [pol1_1] -> lo_1/2(pol1)
  [pol2_1] -> lo_1/3(pol2)
  lo_1/1(pol1_im) -> [pol1_imag_1]
  lo_1/2(pol2_im) -> [pol2_imag_1]
  lo_1/3(pol1_re) -> [pol1_real_1]
  lo_1/4(pol2_re) -> [pol2_real_1]
  [pol1_2] -> lo_2/2(pol1)
  [pol2_2] -> lo_2/3(pol2)
  [pol1_3] -> lo_3/2(pol1)
  [pol2_3] -> lo_3/3(pol2)
  lo_3/1(pol1_im) -> [pol1_imag_3]
  lo_3/2(pol2_im) -> [pol2_imag_3]
  lo_3/3(pol1_re) -> [pol1_real_3]
  lo_3/4(pol2_re) -> [pol2_real_3]
  [mixer_addr] -> lo_5/1(addr)
  [pol1_5] -> lo_5/2(pol1)
  [pol2_5] -> lo_5/3(pol2)
  lo_5/1(pol1_im) -> [pol1_imag_5]
  lo_5/2(pol2_im) -> [pol2_imag_5]
  lo_5/3(pol1_re) -> [pol1_real_5]
  lo_5/4(pol2_re) -> [pol2_real_5]
  [mixer_addr] -> lo_6/1(addr)
  [pol1_6] -> lo_6/2(pol1)
  [pol2_6] -> lo_6/3(pol2)
  lo_6/1(pol1_im) -> [pol1_imag_6]
  lo_6/2(pol2_im) -> [pol2_imag_6]
  lo_6/3(pol1_re) -> [pol1_real_6]
  lo_6/4(pol2_re) -> [pol2_real_6]
  [mixer_addr] -> lo_7/1(addr)
  [pol1_7] -> lo_7/2(pol1)
  [pol2_7] -> lo_7/3(pol2)
  lo_7/1(pol1_im) -> [pol1_imag_7]
  lo_7/2(pol2_im) -> [pol2_imag_7]
  lo_7/3(pol1_re) -> [pol1_real_7]
  lo_7/4(pol2_re) -> [pol2_real_7]
  lo_2/1(pol1_im) -> [pol1_imag_2]
  lo_2/2(pol2_im) -> [pol2_imag_2]
  lo_2/3(pol1_re) -> [pol1_real_2]
  lo_2/4(pol2_re) -> [pol2_real_2]
  [pol1_real_0] -> Gateway Out4/1
  [pol1_imag_0] -> Gateway Out1/1
  [pol2_real_0] -> Gateway Out2/1
  [pol2_imag_0] -> Gateway Out3/1
  Gateway Out4/1 -> Scope/1
  Gateway Out1/1 -> Scope/2
  Gateway Out2/1 -> Scope/3
  Gateway Out3/1 -> Scope/4
  adc_CIC_sync/1 -> [cic_sync]
  Concat4/1 -> mixer_snap64/1(din)
  Constant2/1 -> mixer_snap64/3(we)
  Convert12/1 -> Reinterpret8/1
  [pol1_real_0] -> Convert12/1
  Reinterpret8/1 -> Reinterpret25/1
  Convert13/1 -> Reinterpret9/1
  [pol1_real_1] -> Convert13/1
  Reinterpret9/1 -> Reinterpret16/1
  Convert14/1 -> Reinterpret10/1
  [pol1_real_2] -> Convert14/1
  Reinterpret10/1 -> Reinterpret17/1
  Convert15/1 -> Reinterpret11/1
  [pol1_real_3] -> Convert15/1
  Reinterpret11/1 -> Reinterpret18/1
  Convert16/1 -> Reinterpret12/1
  [pol1_real_4] -> Convert16/1
  Reinterpret12/1 -> Reinterpret19/1
  Convert17/1 -> Reinterpret13/1
  [pol1_real_5] -> Convert17/1
  Reinterpret13/1 -> Reinterpret20/1
  Convert18/1 -> Reinterpret14/1
  [pol1_real_6] -> Convert18/1
  Reinterpret14/1 -> Reinterpret21/1
  Convert19/1 -> Reinterpret15/1
  [pol1_real_7] -> Convert19/1
  Reinterpret15/1 -> Reinterpret22/1
  Reinterpret25/1 -> Concat4/1
  Reinterpret16/1 -> Concat4/2
  Reinterpret17/1 -> Concat4/3
  Reinterpret18/1 -> Concat4/4
  Reinterpret19/1 -> Concat4/5
  Reinterpret20/1 -> Concat4/6
  Reinterpret21/1 -> Concat4/7
  Reinterpret22/1 -> Concat4/8
  Constant5/1 -> trig2/1
  trig2/1 -> Slice2/1
  Slice2/1 -> mixer_snap64/2(trig)
  [pol1_real_quant] -> pol1_re/1
  cic_pow2_0/2(sync_out) -> Halfband4/2(sync)
  cic_stage_dec2/2(sync_out) -> cic_stage_dec1/2(sync_in)
  cic_stage_dec2/1(out) -> cic_stage_dec1/1(in)
  Halfband4/1(out) -> Convert1/1
  [cic_sync] -> cic_stage_dec2/2(sync_in)
  cic_pow2_0/1(out1) -> Convert2/1
  Convert2/1 -> Halfband4/1(in)
  Convert1/1 -> cic_stage_dec2/1(in), [pol1_real_halfband]
  Convert3/1 -> [pol1_real]
  [pol1_imag_quant] -> pol1_im/1
  quant/1(Out1) -> [pol1_real_quant]
  quant/2(Out2) -> [pol1_imag_quant]
  [pol1_real] -> quant/1(In2)
  [pol1_imag] -> quant/2(In3)
  cic_stage_dec4/2(sync_out) -> cic_stage_dec3/2(sync_in)
  cic_stage_dec4/1(out) -> cic_stage_dec3/1(in)
  Halfband1/1(out) -> Convert5/1
  [cic_sync] -> cic_stage_dec4/2(sync_in)
  cic_pow2_1/1(out1) -> Convert4/1
  cic_pow2_1/2(sync_out) -> Halfband1/2(sync)
  Convert4/1 -> Halfband1/1(in)
  Convert5/1 -> cic_stage_dec4/1(in)
  Convert6/1 -> [pol1_imag]
  [pol2_real] -> quant/3(In4)
  [pol2_imag] -> quant/4(In5)
  quant/3(Out3) -> [pol2_real_quant]
  quant/4(Out4) -> [pol2_imag_quant]
  [pol2_real_quant] -> pol2_re/1
  cic_stage_dec6/2(sync_out) -> cic_stage_dec5/2(sync_in)
  cic_stage_dec6/1(out) -> cic_stage_dec5/1(in)
  Halfband2/1(out) -> Convert8/1
  [cic_sync] -> cic_stage_dec6/2(sync_in)
  cic_pow2_2/1(out1) -> Convert7/1
  cic_pow2_2/2(sync_out) -> Halfband2/2(sync)
  Convert7/1 -> Halfband2/1(in)
  Convert8/1 -> cic_stage_dec6/1(in)
  Convert9/1 -> [pol2_real]
  [pol2_imag_quant] -> pol2_im/1
  cic_stage_dec8/2(sync_out) -> cic_stage_dec7/2(sync_in)
  cic_stage_dec8/1(out) -> cic_stage_dec7/1(in)
  Halfband3/1(out) -> Convert11/1
  [cic_sync] -> cic_stage_dec8/2(sync_in)
  cic_pow2_3/1(out1) -> Convert10/1
  cic_pow2_3/2(sync_out) -> Halfband3/2(sync)
  Convert10/1 -> Halfband3/1(in)
  Convert11/1 -> cic_stage_dec8/1(in)
  Convert20/1 -> [pol2_imag]
  pol1_in9/1 -> [pol1_9]
  pol1_in10/1 -> [pol1_10]
  pol1_in11/1 -> [pol1_11]
  pol1_in12/1 -> [pol1_12]
  pol1_in13/1 -> [pol1_13]
  pol1_in14/1 -> [pol1_14]
  pol1_in15/1 -> [pol1_15]
  pol2_in8/1 -> [pol2_8]
  pol2_in9/1 -> [pol2_9]
  pol2_in10/1 -> [pol2_10]
  pol2_in11/1 -> [pol2_11]
  pol2_in12/1 -> [pol2_12]
  pol2_in13/1 -> [pol2_13]
  pol2_in14/1 -> [pol2_14]
  pol2_in15/1 -> [pol2_15]
  [mixer_addr] -> lo_9/1(addr)
  [mixer_addr] -> lo_10/1(addr)
  [mixer_addr] -> lo_11/1(addr)
  lo_12/1(pol1_im) -> [pol1_imag_12]
  lo_12/2(pol2_im) -> [pol2_imag_12]
  lo_12/3(pol1_re) -> [pol1_real_12]
  lo_12/4(pol2_re) -> [pol2_real_12]
  [mixer_addr] -> lo_12/1(addr)
  [pol2_12] -> lo_12/3(pol2)
  [pol1_12] -> lo_12/2(pol1)
  [mixer_addr] -> lo_8/1(addr)
  [pol1_8] -> lo_8/2(pol1)
  [pol2_8] -> lo_8/3(pol2)
  lo_8/1(pol1_im) -> [pol1_imag_8]
  lo_8/2(pol2_im) -> [pol2_imag_8]
  lo_8/3(pol1_re) -> [pol1_real_8]
  lo_8/4(pol2_re) -> [pol2_real_8]
  [pol1_9] -> lo_9/2(pol1)
  [pol2_9] -> lo_9/3(pol2)
  lo_9/1(pol1_im) -> [pol1_imag_9]
  lo_9/2(pol2_im) -> [pol2_imag_9]
  lo_9/3(pol1_re) -> [pol1_real_9]
  lo_9/4(pol2_re) -> [pol2_real_9]
  [pol1_10] -> lo_10/2(pol1)
  [pol2_10] -> lo_10/3(pol2)
  [pol1_11] -> lo_11/2(pol1)
  [pol2_11] -> lo_11/3(pol2)
  lo_11/1(pol1_im) -> [pol1_imag_11]
  lo_11/2(pol2_im) -> [pol2_imag_11]
  lo_11/3(pol1_re) -> [pol1_real_11]
  lo_11/4(pol2_re) -> [pol2_real_11]
  [mixer_addr] -> lo_13/1(addr)
  [pol1_13] -> lo_13/2(pol1)
  [pol2_13] -> lo_13/3(pol2)
  lo_13/1(pol1_im) -> [pol1_imag_13]
  lo_13/2(pol2_im) -> [pol2_imag_13]
  lo_13/3(pol1_re) -> [pol1_real_13]
  lo_13/4(pol2_re) -> [pol2_real_13]
  [mixer_addr] -> lo_14/1(addr)
  [pol1_14] -> lo_14/2(pol1)
  [pol2_14] -> lo_14/3(pol2)
  lo_14/1(pol1_im) -> [pol1_imag_14]
  lo_14/2(pol2_im) -> [pol2_imag_14]
  lo_14/3(pol1_re) -> [pol1_real_14]
  lo_14/4(pol2_re) -> [pol2_real_14]
  [mixer_addr] -> lo_15/1(addr)
  [pol1_15] -> lo_15/2(pol1)
  [pol2_15] -> lo_15/3(pol2)
  lo_15/1(pol1_im) -> [pol1_imag_15]
  lo_15/2(pol2_im) -> [pol2_imag_15]
  lo_15/3(pol1_re) -> [pol1_real_15]
  lo_15/4(pol2_re) -> [pol2_real_15]
  lo_10/1(pol1_im) -> [pol1_imag_10]
  lo_10/2(pol2_im) -> [pol2_imag_10]
  lo_10/3(pol1_re) -> [pol1_real_10]
  lo_10/4(pol2_re) -> [pol2_real_10]
  [cic_sync] -> cic_pow2_0/17(sync_in)
  [pol1_real_0] -> cic_pow2_0/1(in1)
  [pol1_real_1] -> cic_pow2_0/2(in2)
  [pol1_real_2] -> cic_pow2_0/3(in3)
  [pol1_real_3] -> cic_pow2_0/4(in4)
  [pol1_real_4] -> cic_pow2_0/5(in5)
  [pol1_real_5] -> cic_pow2_0/6(in6)
  [pol1_real_6] -> cic_pow2_0/7(in7)
  [pol1_real_7] -> cic_pow2_0/8(in8)
  [pol1_real_8] -> cic_pow2_0/9(in9)
  [pol1_real_9] -> cic_pow2_0/10(in10)
  [pol1_real_10] -> cic_pow2_0/11(in11)
  [pol1_real_11] -> cic_pow2_0/12(in12)
  [pol1_real_12] -> cic_pow2_0/13(in13)
  [pol1_real_13] -> cic_pow2_0/14(in14)
  [pol1_real_14] -> cic_pow2_0/15(in15)
  [pol1_real_15] -> cic_pow2_0/16(in16)
  [cic_sync] -> cic_pow2_3/17(sync_in)
  [pol2_imag_0] -> cic_pow2_3/1(in1)
  [pol2_imag_1] -> cic_pow2_3/2(in2)
  [pol2_imag_2] -> cic_pow2_3/3(in3)
  [pol2_imag_3] -> cic_pow2_3/4(in4)
  [pol2_imag_4] -> cic_pow2_3/5(in5)
  [pol2_imag_5] -> cic_pow2_3/6(in6)
  [pol2_imag_6] -> cic_pow2_3/7(in7)
  [pol2_imag_7] -> cic_pow2_3/8(in8)
  [pol2_imag_8] -> cic_pow2_3/9(in9)
  [pol2_imag_9] -> cic_pow2_3/10(in10)
  [pol2_imag_10] -> cic_pow2_3/11(in11)
  [pol2_imag_11] -> cic_pow2_3/12(in12)
  [pol2_imag_12] -> cic_pow2_3/13(in13)
  [pol2_imag_13] -> cic_pow2_3/14(in14)
  [pol2_imag_14] -> cic_pow2_3/15(in15)
  [pol2_imag_15] -> cic_pow2_3/16(in16)
  [cic_sync] -> cic_pow2_2/17(sync_in)
  [pol2_real_0] -> cic_pow2_2/1(in1)
  [pol2_real_1] -> cic_pow2_2/2(in2)
  [pol2_real_2] -> cic_pow2_2/3(in3)
  [pol2_real_3] -> cic_pow2_2/4(in4)
  [pol2_real_4] -> cic_pow2_2/5(in5)
  [pol2_real_5] -> cic_pow2_2/6(in6)
  [pol2_real_6] -> cic_pow2_2/7(in7)
  [pol2_real_7] -> cic_pow2_2/8(in8)
  [pol2_real_8] -> cic_pow2_2/9(in9)
  [pol2_real_9] -> cic_pow2_2/10(in10)
  [pol2_real_10] -> cic_pow2_2/11(in11)
  [pol2_real_11] -> cic_pow2_2/12(in12)
  [pol2_real_12] -> cic_pow2_2/13(in13)
  [pol2_real_13] -> cic_pow2_2/14(in14)
  [pol2_real_14] -> cic_pow2_2/15(in15)
  [pol2_real_15] -> cic_pow2_2/16(in16)
  [cic_sync] -> cic_pow2_1/17(sync_in)
  [pol1_imag_0] -> cic_pow2_1/1(in1)
  [pol1_imag_1] -> cic_pow2_1/2(in2)
  [pol1_imag_2] -> cic_pow2_1/3(in3)
  [pol1_imag_3] -> cic_pow2_1/4(in4)
  [pol1_imag_4] -> cic_pow2_1/5(in5)
  [pol1_imag_5] -> cic_pow2_1/6(in6)
  [pol1_imag_6] -> cic_pow2_1/7(in7)
  [pol1_imag_7] -> cic_pow2_1/8(in8)
  [pol1_imag_8] -> cic_pow2_1/9(in9)
  [pol1_imag_9] -> cic_pow2_1/10(in10)
  [pol1_imag_10] -> cic_pow2_1/11(in11)
  [pol1_imag_11] -> cic_pow2_1/12(in12)
  [pol1_imag_12] -> cic_pow2_1/13(in13)
  [pol1_imag_13] -> cic_pow2_1/14(in14)
  [pol1_imag_14] -> cic_pow2_1/15(in15)
  [pol1_imag_15] -> cic_pow2_1/16(in16)
  pol1_in8/1 -> [pol1_8]
  cic_stage_dec1/1(out) -> cic_stage_dec11/1(in)
  cic_stage_dec1/2(sync_out) -> cic_stage_dec11/2(sync_in)
  cic_stage_dec11/1(out) -> Convert3/1
  cic_stage_dec3/1(out) -> cic_stage_dec9/1(in)
  cic_stage_dec3/2(sync_out) -> cic_stage_dec9/2(sync_in)
  cic_stage_dec9/1(out) -> Convert6/1
  cic_stage_dec5/1(out) -> cic_stage_dec10/1(in)
  cic_stage_dec5/2(sync_out) -> cic_stage_dec10/2(sync_in)
  cic_stage_dec10/1(out) -> Convert9/1
  cic_stage_dec7/1(out) -> cic_stage_dec12/1(in)
  cic_stage_dec12/1(out) -> Convert20/1
  cic_stage_dec7/2(sync_out) -> cic_stage_dec12/2(sync_in)
